simulink library "Garbage"  (R2023a)
settings: Solver=ode45  StartTime=0.0  StopTime=10.0  RelTol=1e-3  EnableMultiTasking=on
undefined symbols (not defined in the file or in its folder): c, ending_column_index, ending_row_index, framebuffer_16x1, framebuffer_2x2, framebuffer_4x4, framebuffer_8x8, HEIGHT, hot, POS_X, POS_Y, starting_column_index, starting_row_index, WIDTH
libraries: Memory_Blocks_Lib x459, simulink x235, simulink_extras x132, dspmtrx3 x116, Bit_Multiplexers_Lib x67, Bit_Demultiplexers_Lib x49, Byte_Multiplexers_Lib x40, Miscellaneous x38, Computer_Library x17, Decoders_Lib x14, commutil2 x12, Arithmetic_Operations_Lib x8
(part 22 of 26)

== system Garbage/Text_Display_1x16/Byte_Demultiplexer_8x1B ==
  Addr3 : Inport
  Addr2 : Inport  Port=2
  Addr1 : Inport  Port=3
  Addr0 : Inport  Port=4
  Char_In(8B) : Inport  Port=5
  Bus Creator{1..16} (16 alike) : BusCreator  Inputs=8
  Bus Selector1 : BusSelector  OutputSignals=signal1,signal2,signal3,signal4,signal5,signal6,signal7,signal8
  Byte_Demultiplexer_1_to_16(0) : Byte_Demultiplexers_Lib/Byte_Demultiplexers_Lib/Byte_Demultiplexer_1_to_16
  Byte_Demultiplexer_1_to_16(1) : Byte_Demultiplexers_Lib/Byte_Demultiplexers_Lib/Byte_Demultiplexer_1_to_16
  Byte_Demultiplexer_1_to_16(2) : Byte_Demultiplexers_Lib/Byte_Demultiplexers_Lib/Byte_Demultiplexer_1_to_16
  Byte_Demultiplexer_1_to_16(3) : Byte_Demultiplexers_Lib/Byte_Demultiplexers_Lib/Byte_Demultiplexer_1_to_16
  Byte_Demultiplexer_1_to_16(4) : Byte_Demultiplexers_Lib/Byte_Demultiplexers_Lib/Byte_Demultiplexer_1_to_16
  Byte_Demultiplexer_1_to_16(5) : Byte_Demultiplexers_Lib/Byte_Demultiplexers_Lib/Byte_Demultiplexer_1_to_16
  Byte_Demultiplexer_1_to_16(6) : Byte_Demultiplexers_Lib/Byte_Demultiplexers_Lib/Byte_Demultiplexer_1_to_16
  Byte_Demultiplexer_1_to_16(7) : Byte_Demultiplexers_Lib/Byte_Demultiplexers_Lib/Byte_Demultiplexer_1_to_16
  Char_Out_15(8B) : Outport
  Char_Out_14(8B) : Outport  Port=2
  Char_Out_13(8B) : Outport  Port=3
  Char_Out_12(8B) : Outport  Port=4
  Char_Out_11(8B) : Outport  Port=5
  Char_Out_10(8B) : Outport  Port=6
  Char_Out_09(8B) : Outport  Port=7
  Char_Out_08(8B) : Outport  Port=8
  Char_Out_07(8B) : Outport  Port=9
  Char_Out_06(8B) : Outport  Port=10
  Char_Out_05(8B) : Outport  Port=11
  Char_Out_04(8B) : Outport  Port=12
  Char_Out_03(8B) : Outport  Port=13
  Char_Out_02(8B) : Outport  Port=14
  Char_Out_01(8B) : Outport  Port=15
  Char_Out_00(8B) : Outport  Port=16
  wires:
  Byte_Demultiplexer_1_to_16(7)/1 -> Bus Creator15/1
  Byte_Demultiplexer_1_to_16(6)/1 -> Bus Creator15/2
  Byte_Demultiplexer_1_to_16(5)/1 -> Bus Creator15/3
  Byte_Demultiplexer_1_to_16(4)/1 -> Bus Creator15/4
  Byte_Demultiplexer_1_to_16(3)/1 -> Bus Creator15/5
  Byte_Demultiplexer_1_to_16(2)/1 -> Bus Creator15/6
  Byte_Demultiplexer_1_to_16(1)/1 -> Bus Creator15/7
  Byte_Demultiplexer_1_to_16(0)/1 -> Bus Creator15/8
  Byte_Demultiplexer_1_to_16(7)/2 -> Bus Creator1/1
  Byte_Demultiplexer_1_to_16(7)/3 -> Bus Creator2/1
  Byte_Demultiplexer_1_to_16(7)/4 -> Bus Creator3/1
  Byte_Demultiplexer_1_to_16(7)/5 -> Bus Creator4/1
  Byte_Demultiplexer_1_to_16(7)/6 -> Bus Creator5/1
  Byte_Demultiplexer_1_to_16(7)/7 -> Bus Creator6/1
  Byte_Demultiplexer_1_to_16(7)/8 -> Bus Creator7/1
  Byte_Demultiplexer_1_to_16(6)/2 -> Bus Creator1/2
  Byte_Demultiplexer_1_to_16(6)/3 -> Bus Creator2/2
  Byte_Demultiplexer_1_to_16(6)/4 -> Bus Creator3/2
  Byte_Demultiplexer_1_to_16(6)/5 -> Bus Creator4/2
  Byte_Demultiplexer_1_to_16(6)/6 -> Bus Creator5/2
  Byte_Demultiplexer_1_to_16(6)/7 -> Bus Creator6/2
  Byte_Demultiplexer_1_to_16(6)/8 -> Bus Creator7/2
  Byte_Demultiplexer_1_to_16(5)/2 -> Bus Creator1/3
  Byte_Demultiplexer_1_to_16(5)/3 -> Bus Creator2/3
  Byte_Demultiplexer_1_to_16(5)/4 -> Bus Creator3/3
  Byte_Demultiplexer_1_to_16(5)/5 -> Bus Creator4/3
  Byte_Demultiplexer_1_to_16(5)/6 -> Bus Creator5/3
  Byte_Demultiplexer_1_to_16(5)/7 -> Bus Creator6/3
  Byte_Demultiplexer_1_to_16(5)/8 -> Bus Creator7/3
  Byte_Demultiplexer_1_to_16(4)/2 -> Bus Creator1/4
  Byte_Demultiplexer_1_to_16(4)/3 -> Bus Creator2/4
  Byte_Demultiplexer_1_to_16(4)/4 -> Bus Creator3/4
  Byte_Demultiplexer_1_to_16(4)/5 -> Bus Creator4/4
  Byte_Demultiplexer_1_to_16(4)/6 -> Bus Creator5/4
  Byte_Demultiplexer_1_to_16(4)/7 -> Bus Creator6/4
  Byte_Demultiplexer_1_to_16(4)/8 -> Bus Creator7/4
  Byte_Demultiplexer_1_to_16(3)/2 -> Bus Creator1/5
  Byte_Demultiplexer_1_to_16(3)/3 -> Bus Creator2/5
  Byte_Demultiplexer_1_to_16(3)/4 -> Bus Creator3/5
  Byte_Demultiplexer_1_to_16(3)/5 -> Bus Creator4/5
  Byte_Demultiplexer_1_to_16(3)/6 -> Bus Creator5/5
  Byte_Demultiplexer_1_to_16(3)/7 -> Bus Creator6/5
  Byte_Demultiplexer_1_to_16(3)/8 -> Bus Creator7/5
  Byte_Demultiplexer_1_to_16(2)/2 -> Bus Creator1/6
  Byte_Demultiplexer_1_to_16(2)/3 -> Bus Creator2/6
  Byte_Demultiplexer_1_to_16(2)/4 -> Bus Creator3/6
  Byte_Demultiplexer_1_to_16(2)/5 -> Bus Creator4/6
  Byte_Demultiplexer_1_to_16(2)/6 -> Bus Creator5/6
  Byte_Demultiplexer_1_to_16(2)/7 -> Bus Creator6/6
  Byte_Demultiplexer_1_to_16(2)/8 -> Bus Creator7/6
  Byte_Demultiplexer_1_to_16(1)/2 -> Bus Creator1/7
  Byte_Demultiplexer_1_to_16(1)/3 -> Bus Creator2/7
  Byte_Demultiplexer_1_to_16(1)/4 -> Bus Creator3/7
  Byte_Demultiplexer_1_to_16(1)/5 -> Bus Creator4/7
  Byte_Demultiplexer_1_to_16(1)/6 -> Bus Creator5/7
  Byte_Demultiplexer_1_to_16(1)/7 -> Bus Creator6/7
  Byte_Demultiplexer_1_to_16(1)/8 -> Bus Creator7/7
  Byte_Demultiplexer_1_to_16(0)/2 -> Bus Creator1/8
  Byte_Demultiplexer_1_to_16(0)/3 -> Bus Creator2/8
  Byte_Demultiplexer_1_to_16(0)/4 -> Bus Creator3/8
  Byte_Demultiplexer_1_to_16(0)/5 -> Bus Creator4/8
  Byte_Demultiplexer_1_to_16(0)/6 -> Bus Creator5/8
  Byte_Demultiplexer_1_to_16(0)/7 -> Bus Creator6/8
  Byte_Demultiplexer_1_to_16(0)/8 -> Bus Creator7/8
  Bus Creator7/1 -> Char_Out_08(8B)/1
  Bus Creator6/1 -> Char_Out_09(8B)/1
  Bus Creator5/1 -> Char_Out_10(8B)/1
  Bus Creator4/1 -> Char_Out_11(8B)/1
  Bus Creator3/1 -> Char_Out_12(8B)/1
  Bus Creator2/1 -> Char_Out_13(8B)/1
  Bus Creator1/1 -> Char_Out_14(8B)/1
  Bus Creator15/1 -> Char_Out_15(8B)/1
  Addr2/1 -> Byte_Demultiplexer_1_to_16(6)/2, Byte_Demultiplexer_1_to_16(5)/2, Byte_Demultiplexer_1_to_16(4)/2, Byte_Demultiplexer_1_to_16(3)/2, Byte_Demultiplexer_1_to_16(0)/2, Byte_Demultiplexer_1_to_16(1)/2, Byte_Demultiplexer_1_to_16(2)/2, Byte_Demultiplexer_1_to_16(7)/2
  Addr1/1 -> Byte_Demultiplexer_1_to_16(7)/3, Byte_Demultiplexer_1_to_16(6)/3, Byte_Demultiplexer_1_to_16(5)/3, Byte_Demultiplexer_1_to_16(4)/3, Byte_Demultiplexer_1_to_16(3)/3, Byte_Demultiplexer_1_to_16(0)/3, Byte_Demultiplexer_1_to_16(1)/3, Byte_Demultiplexer_1_to_16(2)/3
  Addr0/1 -> Byte_Demultiplexer_1_to_16(7)/4, Byte_Demultiplexer_1_to_16(6)/4, Byte_Demultiplexer_1_to_16(5)/4, Byte_Demultiplexer_1_to_16(3)/4, Byte_Demultiplexer_1_to_16(0)/4, Byte_Demultiplexer_1_to_16(1)/4, Byte_Demultiplexer_1_to_16(2)/4, Byte_Demultiplexer_1_to_16(4)/4
  Byte_Demultiplexer_1_to_16(4)/9 -> Bus Creator9/4
  Byte_Demultiplexer_1_to_16(3)/9 -> Bus Creator9/5
  Byte_Demultiplexer_1_to_16(2)/9 -> Bus Creator9/6
  Byte_Demultiplexer_1_to_16(1)/9 -> Bus Creator9/7
  Byte_Demultiplexer_1_to_16(0)/9 -> Bus Creator9/8
  Byte_Demultiplexer_1_to_16(7)/10 -> Bus Creator8/1
  Byte_Demultiplexer_1_to_16(7)/11 -> Bus Creator10/1
  Byte_Demultiplexer_1_to_16(7)/12 -> Bus Creator11/1
  Byte_Demultiplexer_1_to_16(7)/13 -> Bus Creator12/1
  Byte_Demultiplexer_1_to_16(7)/14 -> Bus Creator13/1
  Byte_Demultiplexer_1_to_16(7)/15 -> Bus Creator14/1
  Byte_Demultiplexer_1_to_16(7)/16 -> Bus Creator16/1
  Byte_Demultiplexer_1_to_16(6)/10 -> Bus Creator8/2
  Byte_Demultiplexer_1_to_16(6)/11 -> Bus Creator10/2
  Byte_Demultiplexer_1_to_16(6)/12 -> Bus Creator11/2
  Byte_Demultiplexer_1_to_16(6)/13 -> Bus Creator12/2
  Byte_Demultiplexer_1_to_16(6)/14 -> Bus Creator13/2
  Byte_Demultiplexer_1_to_16(6)/15 -> Bus Creator14/2
  Byte_Demultiplexer_1_to_16(6)/16 -> Bus Creator16/2
  Byte_Demultiplexer_1_to_16(5)/10 -> Bus Creator8/3
  Byte_Demultiplexer_1_to_16(5)/11 -> Bus Creator10/3
  Byte_Demultiplexer_1_to_16(5)/12 -> Bus Creator11/3
  Byte_Demultiplexer_1_to_16(5)/13 -> Bus Creator12/3
  Byte_Demultiplexer_1_to_16(5)/14 -> Bus Creator13/3
  Byte_Demultiplexer_1_to_16(5)/15 -> Bus Creator14/3
  Byte_Demultiplexer_1_to_16(5)/16 -> Bus Creator16/3
  Byte_Demultiplexer_1_to_16(4)/10 -> Bus Creator8/4
  Byte_Demultiplexer_1_to_16(4)/11 -> Bus Creator10/4
  Byte_Demultiplexer_1_to_16(4)/12 -> Bus Creator11/4
  Byte_Demultiplexer_1_to_16(4)/13 -> Bus Creator12/4
  Byte_Demultiplexer_1_to_16(4)/14 -> Bus Creator13/4
  Byte_Demultiplexer_1_to_16(4)/15 -> Bus Creator14/4
  Byte_Demultiplexer_1_to_16(4)/16 -> Bus Creator16/4
  Byte_Demultiplexer_1_to_16(3)/10 -> Bus Creator8/5
  Byte_Demultiplexer_1_to_16(3)/11 -> Bus Creator10/5
  Byte_Demultiplexer_1_to_16(3)/12 -> Bus Creator11/5
  Byte_Demultiplexer_1_to_16(3)/13 -> Bus Creator12/5
  Byte_Demultiplexer_1_to_16(3)/14 -> Bus Creator13/5
  Byte_Demultiplexer_1_to_16(3)/15 -> Bus Creator14/5
  Byte_Demultiplexer_1_to_16(3)/16 -> Bus Creator16/5
  Byte_Demultiplexer_1_to_16(2)/10 -> Bus Creator8/6
  Byte_Demultiplexer_1_to_16(2)/11 -> Bus Creator10/6
  Byte_Demultiplexer_1_to_16(2)/12 -> Bus Creator11/6
  Byte_Demultiplexer_1_to_16(2)/13 -> Bus Creator12/6
  Byte_Demultiplexer_1_to_16(2)/14 -> Bus Creator13/6
  Byte_Demultiplexer_1_to_16(2)/15 -> Bus Creator14/6
  Byte_Demultiplexer_1_to_16(2)/16 -> Bus Creator16/6
  Byte_Demultiplexer_1_to_16(1)/10 -> Bus Creator8/7
  Byte_Demultiplexer_1_to_16(1)/11 -> Bus Creator10/7
  Byte_Demultiplexer_1_to_16(1)/12 -> Bus Creator11/7
  Byte_Demultiplexer_1_to_16(1)/13 -> Bus Creator12/7
  Byte_Demultiplexer_1_to_16(1)/14 -> Bus Creator13/7
  Byte_Demultiplexer_1_to_16(1)/15 -> Bus Creator14/7
  Byte_Demultiplexer_1_to_16(1)/16 -> Bus Creator16/7
  Byte_Demultiplexer_1_to_16(0)/10 -> Bus Creator8/8
  Byte_Demultiplexer_1_to_16(0)/11 -> Bus Creator10/8
  Byte_Demultiplexer_1_to_16(0)/12 -> Bus Creator11/8
  Byte_Demultiplexer_1_to_16(0)/13 -> Bus Creator12/8
  Byte_Demultiplexer_1_to_16(0)/14 -> Bus Creator13/8
  Byte_Demultiplexer_1_to_16(0)/15 -> Bus Creator14/8
  Byte_Demultiplexer_1_to_16(0)/16 -> Bus Creator16/8
  Bus Creator16/1 -> Char_Out_00(8B)/1
  Bus Creator14/1 -> Char_Out_01(8B)/1
  Bus Creator13/1 -> Char_Out_02(8B)/1
  Bus Creator12/1 -> Char_Out_03(8B)/1
  Bus Creator11/1 -> Char_Out_04(8B)/1
  Bus Creator10/1 -> Char_Out_05(8B)/1
  Bus Creator8/1 -> Char_Out_06(8B)/1
  Bus Creator9/1 -> Char_Out_07(8B)/1
  Byte_Demultiplexer_1_to_16(5)/9 -> Bus Creator9/3
  Byte_Demultiplexer_1_to_16(6)/9 -> Bus Creator9/2
  Byte_Demultiplexer_1_to_16(7)/9 -> Bus Creator9/1
  Addr3/1 -> Byte_Demultiplexer_1_to_16(5)/1, Byte_Demultiplexer_1_to_16(4)/1, Byte_Demultiplexer_1_to_16(3)/1, Byte_Demultiplexer_1_to_16(2)/1, Byte_Demultiplexer_1_to_16(1)/1, Byte_Demultiplexer_1_to_16(0)/1, Byte_Demultiplexer_1_to_16(6)/1, Byte_Demultiplexer_1_to_16(7)/1
  Char_In(8B)/1 -> Bus Selector1/1
  Bus Selector1/1 -> Byte_Demultiplexer_1_to_16(7)/5  "<signal1>"
  Bus Selector1/2 -> Byte_Demultiplexer_1_to_16(6)/5  "<signal2>"
  Bus Selector1/3 -> Byte_Demultiplexer_1_to_16(5)/5  "<signal3>"
  Bus Selector1/4 -> Byte_Demultiplexer_1_to_16(4)/5  "<signal4>"
  Bus Selector1/5 -> Byte_Demultiplexer_1_to_16(3)/5  "<signal5>"
  Bus Selector1/6 -> Byte_Demultiplexer_1_to_16(2)/5  "<signal6>"
  Bus Selector1/7 -> Byte_Demultiplexer_1_to_16(1)/5  "<signal7>"
  Bus Selector1/8 -> Byte_Demultiplexer_1_to_16(0)/5  "<signal8>"

== system Garbage/Text_Display_1x16/Character_Table ==
  Address_In(1B) : Inport
  Bus Creator : BusCreator  Inputs=8
  Bus Selector1 : BusSelector  OutputSignals=signal11,signal12,signal13,signal14,signal15,signal16
  Byte_Multiplexer_4_to_1(Byte_0) : Byte_Multiplexers_Lib/Byte_Multiplexers_Lib/Byte_Multiplexer_4_to_1
  Byte_Multiplexer_4_to_1(Byte_1) : Byte_Multiplexers_Lib/Byte_Multiplexers_Lib/Byte_Multiplexer_4_to_1
  Byte_Multiplexer_4_to_1(Byte_2) : Byte_Multiplexers_Lib/Byte_Multiplexers_Lib/Byte_Multiplexer_4_to_1
  Byte_Multiplexer_4_to_1(Byte_3) : Byte_Multiplexers_Lib/Byte_Multiplexers_Lib/Byte_Multiplexer_4_to_1
  Byte_Multiplexer_4_to_1(Byte_4) : Byte_Multiplexers_Lib/Byte_Multiplexers_Lib/Byte_Multiplexer_4_to_1
  Byte_Multiplexer_4_to_1(Byte_5) : Byte_Multiplexers_Lib/Byte_Multiplexers_Lib/Byte_Multiplexer_4_to_1
  Byte_Multiplexer_4_to_1(Byte_6) : Byte_Multiplexers_Lib/Byte_Multiplexers_Lib/Byte_Multiplexer_4_to_1
  Byte_Multiplexer_4_to_1(Byte_7) : Byte_Multiplexers_Lib/Byte_Multiplexers_Lib/Byte_Multiplexer_4_to_1
  Characters(0..F) : Subsystem
  Characters(ARROW_DOWN..RECTANGLE) : Subsystem
  Characters(G..W) : Subsystem
  Characters(Q..ARROW_UP) : Subsystem
  Data_Out(8x1B) : Outport
  wires:
  Bus Creator/1 -> Data_Out(8x1B)/1
  Address_In(1B)/1 -> Bus Selector1/1
  Byte_Multiplexer_4_to_1(Byte_3)/1 -> Bus Creator/5
  Bus Selector1/5 -> Characters(0..F)/3(Address_1_In(1b)), Characters(G..W)/3(Address_1_In(1b)), Characters(Q..ARROW_UP)/3(Address_1_In(1b)), Characters(ARROW_DOWN..RECTANGLE)/3(Address_1_In(1b))  "<signal15>"
  Byte_Multiplexer_4_to_1(Byte_7)/1 -> Bus Creator/1
  Byte_Multiplexer_4_to_1(Byte_0)/1 -> Bus Creator/8
  Byte_Multiplexer_4_to_1(Byte_4)/1 -> Bus Creator/4
  Byte_Multiplexer_4_to_1(Byte_5)/1 -> Bus Creator/3
  Byte_Multiplexer_4_to_1(Byte_2)/1 -> Bus Creator/6
  Byte_Multiplexer_4_to_1(Byte_6)/1 -> Bus Creator/2
  Bus Selector1/3 -> Characters(0..F)/1(Address_3_In(1b)), Characters(G..W)/1(Address_3_In(1b)), Characters(Q..ARROW_UP)/1(Address_3_In(1b)), Characters(ARROW_DOWN..RECTANGLE)/1(Address_3_In(1b))  "<signal13>"
  Byte_Multiplexer_4_to_1(Byte_1)/1 -> Bus Creator/7
  Bus Selector1/4 -> Characters(0..F)/2(Address_2_In(1b)), Characters(G..W)/2(Address_2_In(1b)), Characters(Q..ARROW_UP)/2(Address_2_In(1b)), Characters(ARROW_DOWN..RECTANGLE)/2(Address_2_In(1b))  "<signal14>"
  Bus Selector1/6 -> Characters(0..F)/4(Address_0_In(1b)), Characters(G..W)/4(Address_0_In(1b)), Characters(Q..ARROW_UP)/4(Address_0_In(1b)), Characters(ARROW_DOWN..RECTANGLE)/4(Address_0_In(1b))  "<signal16>"
  Characters(ARROW_DOWN..RECTANGLE)/4(Row_4(1B)) -> Byte_Multiplexer_4_to_1(Byte_4)/3
  Characters(ARROW_DOWN..RECTANGLE)/1(Row_7(1B)) -> Byte_Multiplexer_4_to_1(Byte_7)/3
  Characters(0..F)/6(Row_2(1B)) -> Byte_Multiplexer_4_to_1(Byte_2)/6
  Characters(G..W)/5(Row_3(1B)) -> Byte_Multiplexer_4_to_1(Byte_3)/5
  Characters(0..F)/5(Row_3(1B)) -> Byte_Multiplexer_4_to_1(Byte_3)/6
  Characters(G..W)/1(Row_7(1B)) -> Byte_Multiplexer_4_to_1(Byte_7)/5
  Characters(0..F)/1(Row_7(1B)) -> Byte_Multiplexer_4_to_1(Byte_7)/6
  Bus Selector1/2 -> Byte_Multiplexer_4_to_1(Byte_7)/2, Byte_Multiplexer_4_to_1(Byte_0)/2, Byte_Multiplexer_4_to_1(Byte_1)/2, Byte_Multiplexer_4_to_1(Byte_2)/2, Byte_Multiplexer_4_to_1(Byte_3)/2, Byte_Multiplexer_4_to_1(Byte_4)/2, Byte_Multiplexer_4_to_1(Byte_5)/2, Byte_Multiplexer_4_to_1(Byte_6)/2  "<signal12>"
  Characters(Q..ARROW_UP)/4(Row_4(1B)) -> Byte_Multiplexer_4_to_1(Byte_4)/4
  Bus Selector1/1 -> Byte_Multiplexer_4_to_1(Byte_7)/1, Byte_Multiplexer_4_to_1(Byte_4)/1, Byte_Multiplexer_4_to_1(Byte_0)/1, Byte_Multiplexer_4_to_1(Byte_1)/1, Byte_Multiplexer_4_to_1(Byte_2)/1, Byte_Multiplexer_4_to_1(Byte_3)/1, Byte_Multiplexer_4_to_1(Byte_5)/1, Byte_Multiplexer_4_to_1(Byte_6)/1  "<signal11>"
  Characters(G..W)/8(Row_0(1B)) -> Byte_Multiplexer_4_to_1(Byte_0)/5
  Characters(ARROW_DOWN..RECTANGLE)/5(Row_3(1B)) -> Byte_Multiplexer_4_to_1(Byte_3)/3
  Characters(Q..ARROW_UP)/8(Row_0(1B)) -> Byte_Multiplexer_4_to_1(Byte_0)/4
  Characters(0..F)/8(Row_0(1B)) -> Byte_Multiplexer_4_to_1(Byte_0)/6
  Characters(G..W)/7(Row_1(1B)) -> Byte_Multiplexer_4_to_1(Byte_1)/5
  Characters(0..F)/7(Row_1(1B)) -> Byte_Multiplexer_4_to_1(Byte_1)/6
  Characters(G..W)/6(Row_2(1B)) -> Byte_Multiplexer_4_to_1(Byte_2)/5
  Characters(Q..ARROW_UP)/3(Row_5(1B)) -> Byte_Multiplexer_4_to_1(Byte_5)/4
  Characters(ARROW_DOWN..RECTANGLE)/3(Row_5(1B)) -> Byte_Multiplexer_4_to_1(Byte_5)/3
  Characters(Q..ARROW_UP)/1(Row_7(1B)) -> Byte_Multiplexer_4_to_1(Byte_7)/4
  Characters(0..F)/4(Row_4(1B)) -> Byte_Multiplexer_4_to_1(Byte_4)/6
  Characters(Q..ARROW_UP)/5(Row_3(1B)) -> Byte_Multiplexer_4_to_1(Byte_3)/4
  Characters(G..W)/3(Row_5(1B)) -> Byte_Multiplexer_4_to_1(Byte_5)/5
  Characters(ARROW_DOWN..RECTANGLE)/6(Row_2(1B)) -> Byte_Multiplexer_4_to_1(Byte_2)/3
  Characters(G..W)/4(Row_4(1B)) -> Byte_Multiplexer_4_to_1(Byte_4)/5
  Characters(ARROW_DOWN..RECTANGLE)/7(Row_1(1B)) -> Byte_Multiplexer_4_to_1(Byte_0)/3
  Characters(G..W)/2(Row_6(1B)) -> Byte_Multiplexer_4_to_1(Byte_6)/5
  Characters(ARROW_DOWN..RECTANGLE)/8(Row_0(1B)) -> Byte_Multiplexer_4_to_1(Byte_1)/3
  Characters(Q..ARROW_UP)/2(Row_6(1B)) -> Byte_Multiplexer_4_to_1(Byte_6)/4
  Characters(0..F)/2(Row_6(1B)) -> Byte_Multiplexer_4_to_1(Byte_6)/6
  Characters(ARROW_DOWN..RECTANGLE)/2(Row_6(1B)) -> Byte_Multiplexer_4_to_1(Byte_6)/3
  Characters(Q..ARROW_UP)/7(Row_1(1B)) -> Byte_Multiplexer_4_to_1(Byte_1)/4
  Characters(Q..ARROW_UP)/6(Row_2(1B)) -> Byte_Multiplexer_4_to_1(Byte_2)/4
  Characters(0..F)/3(Row_5(1B)) -> Byte_Multiplexer_4_to_1(Byte_5)/6

== system Garbage/Text_Display_1x16/Counter_4bit ==
  Clock : Inport
  Bit_{0..3} (4 alike) : simulink_extras/Flip Flops/J-K Flip-Flop  initial_condition=0
  Constant8 : Constant  OutDataTypeStr=boolean
  Terminator{1..2,10,12} (4 alike) : Terminator
  Addr_3(1b) : Outport
  Addr_2(1b) : Outport  Port=2
  Addr_1(1b) : Outport  Port=3
  Addr_0(1b) : Outport  Port=4
  wires:
  Clock/1 -> Bit_0/2
  Bit_0/2 -> Terminator10/1
  Bit_3/2 -> Terminator12/1
  Bit_3/1 -> Addr_3(1b)/1
  Constant8/1 -> Bit_0/1, Bit_0/3
  Bit_0/1 -> Addr_0(1b)/1, Bit_1/1, Bit_1/2, Bit_1/3
  Bit_1/1 -> Addr_1(1b)/1, Bit_2/1, Bit_2/2, Bit_2/3
  Bit_1/2 -> Terminator1/1
  Bit_2/2 -> Terminator2/1
  Bit_2/1 -> Addr_2(1b)/1, Bit_3/1, Bit_3/2, Bit_3/3
